simulink model "SdbTxSigProc"  (R2022b) (continued)
== system SdbTxSigProc == (continued)
  HvacAirTReAtFlrLeRaw_HvacAirTReAtFlrLeRaw/1 -> SdbTxSigProc_Runnable_sys/168(HvacAirTReAtFlrLeRaw_HvacAirTReAtFlrLeRaw_read)
  HvacAirTReAtVentLeRaw_HvacAirTReAtVentLeRaw/1 -> SdbTxSigProc_Runnable_sys/169(HvacAirTReAtVentLeRaw_HvacAirTReAtVentLeRaw_read)
  HvacEvaprTSpReNgcc_HvacEvaprTSpReNgcc/1 -> SdbTxSigProc_Runnable_sys/170(HvacEvaprTSpReNgcc_HvacEvaprTSpReNgcc_read)
  HvacFanLvlFbNgcc_HvacFanLvlFbNgcc/1 -> SdbTxSigProc_Runnable_sys/171(HvacFanLvlFbNgcc_HvacFanLvlFbNgcc_read)
  HvacFanLvlFbReNgcc_HvacFanLvlFbReNgcc/1 -> SdbTxSigProc_Runnable_sys/172(HvacFanLvlFbReNgcc_HvacFanLvlFbReNgcc_read)
  HvacHeatElmAirMFlowEstimdFirstLeNgcc_HvacHeatElmAirMFlowEstimdFirstLeNgcc/1 -> SdbTxSigProc_Runnable_sys/173(HvacHeatElmAirMFlowEstimdFirstLeNgcc_HvacHeatElmAirMFlowEstimdFirstLeNgcc_read)
  HvacHeatElmAirMFlowEstimdFirstRiNgcc_HvacHeatElmAirMFlowEstimdFirstRiNgcc/1 -> SdbTxSigProc_Runnable_sys/174(HvacHeatElmAirMFlowEstimdFirstRiNgcc_HvacHeatElmAirMFlowEstimdFirstRiNgcc_read)
  HvacHeatElmAirMFlowEstimdSecLeNgcc_HvacHeatElmAirMFlowEstimdSecLeNgcc/1 -> SdbTxSigProc_Runnable_sys/175(HvacHeatElmAirMFlowEstimdSecLeNgcc_HvacHeatElmAirMFlowEstimdSecLeNgcc_read)
  HvacHeatgReqReInt_HvacHeatgReqReInt/1 -> SdbTxSigProc_Runnable_sys/176(HvacHeatgReqReInt_HvacHeatgReqReInt_read)
  HvacHexAirTForRowFirstLeRaw_HvacHexAirTForRowFirstLeRaw/1 -> SdbTxSigProc_Runnable_sys/177(HvacHexAirTForRowFirstLeRaw_HvacHexAirTForRowFirstLeRaw_read)
  HvacHexAirTForRowFirstRiRaw_HvacHexAirTForRowFirstRiRaw/1 -> SdbTxSigProc_Runnable_sys/178(HvacHexAirTForRowFirstRiRaw_HvacHexAirTForRowFirstRiRaw_read)
  HvacHexAirTForRowSecLeRaw_HvacHexAirTForRowSecLeRaw/1 -> SdbTxSigProc_Runnable_sys/179(HvacHexAirTForRowSecLeRaw_HvacHexAirTForRowSecLeRaw_read)
  KeySynchrnztnLclNgcc_KeySynchrnztnLclNgcc/1 -> SdbTxSigProc_Runnable_sys/180(KeySynchrnztnLclNgcc_KeySynchrnztnLclNgcc_read)
  OutlSovActvStsNgcc_OutlSovActvStsNgcc/1 -> SdbTxSigProc_Runnable_sys/181(OutlSovActvStsNgcc_OutlSovActvStsNgcc_read)
  OxygenConcSnsrActnReqNgcc_OxygenConcSnsrActnReqNgcc/1 -> SdbTxSigProc_Runnable_sys/182(OxygenConcSnsrActnReqNgcc_OxygenConcSnsrActnReqNgcc_read)
  OxygenConcSnsrStsFrmClimaNgcc_OxygenConcSnsrStsFrmClimaNgcc/1 -> SdbTxSigProc_Runnable_sys/183(OxygenConcSnsrStsFrmClimaNgcc_OxygenConcSnsrStsFrmClimaNgcc_read)
  OxygenSplyAvlStsFrmClimaNgcc_OxygenSplyAvlStsFrmClimaNgcc/1 -> SdbTxSigProc_Runnable_sys/184(OxygenSplyAvlStsFrmClimaNgcc_OxygenSplyAvlStsFrmClimaNgcc_read)
  OxygenSplyErrFrmClimaNgcc_OxygenSplyErrFrmClimaNgcc/1 -> SdbTxSigProc_Runnable_sys/185(OxygenSplyErrFrmClimaNgcc_OxygenSplyErrFrmClimaNgcc_read)
  OxygenSplyPopUpReqNgcc_OxygenSplyPopUpReqNgcc/1 -> SdbTxSigProc_Runnable_sys/186(OxygenSplyPopUpReqNgcc_OxygenSplyPopUpReqNgcc_read)
  OxygenSplyPwrAllwdNgcc_OxygenSplyPwrAllwdNgcc/1 -> SdbTxSigProc_Runnable_sys/187(OxygenSplyPwrAllwdNgcc_OxygenSplyPwrAllwdNgcc_read)
  OxygenSplyReqFaildNgcc_OxygenSplyReqFaildNgcc/1 -> SdbTxSigProc_Runnable_sys/188(OxygenSplyReqFaildNgcc_OxygenSplyReqFaildNgcc_read)
  OxygenSplyReqNgcc_OxygenSplyReqNgcc/1 -> SdbTxSigProc_Runnable_sys/189(OxygenSplyReqNgcc_OxygenSplyReqNgcc_read)
  OxygenSplyVoiceFbNgcc_OxygenSplyVoiceFbNgcc/1 -> SdbTxSigProc_Runnable_sys/190(OxygenSplyVoiceFbNgcc_OxygenSplyVoiceFbNgcc_read)
  PrkgClimaEngStReqNgcc_PrkgClimaEngStReqNgcc/1 -> SdbTxSigProc_Runnable_sys/191(PrkgClimaEngStReqNgcc_PrkgClimaEngStReqNgcc_read)
  PwrCnsForHvacFanFrntNgcc_PwrCnsForHvacFanFrnt/1 -> SdbTxSigProc_Runnable_sys/192(PwrCnsForHvacFanFrntNgcc_PwrCnsForHvacFanFrnt_read)
  PwrCnsForHvacFanReNgcc_PwrCnsForHvacFanRe/1 -> SdbTxSigProc_Runnable_sys/193(PwrCnsForHvacFanReNgcc_PwrCnsForHvacFanRe_read)
  ReClimaManModCmftStsNgcc_ReClimaManModCmftStsNgcc/1 -> SdbTxSigProc_Runnable_sys/194(ReClimaManModCmftStsNgcc_ReClimaManModCmftStsNgcc_read)
  RemOxygenSplyActvFaildNgcc_RemOxygenSplyActvFaildNgcc/1 -> SdbTxSigProc_Runnable_sys/195(RemOxygenSplyActvFaildNgcc_RemOxygenSplyActvFaildNgcc_read)
  TCSCmprStrtFltNgcc_TCSCmprStrtFltNgcc/1 -> SdbTxSigProc_Runnable_sys/196(TCSCmprStrtFltNgcc_TCSCmprStrtFltNgcc_read)
  TCScoolgGrdReqNgcc_TCScoolgGrdReqNgcc/1 -> SdbTxSigProc_Runnable_sys/197(TCScoolgGrdReqNgcc_TCScoolgGrdReqNgcc_read)
  TCSCooltFlowReq1Ngcc_TCSCooltFlowReq1Ngcc/1 -> SdbTxSigProc_Runnable_sys/198(TCSCooltFlowReq1Ngcc_TCSCooltFlowReq1Ngcc_read)
  TCSCooltTReq1Ngcc_TCSCooltTReq1Ngcc/1 -> SdbTxSigProc_Runnable_sys/199(TCSCooltTReq1Ngcc_TCSCooltTReq1Ngcc_read)
  TCSDoorStsNgcc_TCSDoorStsNgcc/1 -> SdbTxSigProc_Runnable_sys/200(TCSDoorStsNgcc_TCSDoorStsNgcc_read)
  TCSDoorTopSts1Ngcc_TCSDoorTopSts1Ngcc/1 -> SdbTxSigProc_Runnable_sys/201(TCSDoorTopSts1Ngcc_TCSDoorTopSts1Ngcc_read)
  TcsEcoModSts1Ngcc_TcsEcoModSts1Ngcc/1 -> SdbTxSigProc_Runnable_sys/202(TcsEcoModSts1Ngcc_TcsEcoModSts1Ngcc_read)
  TcsFltNewSts1Ngcc_TcsFltNewSts1Ngcc/1 -> SdbTxSigProc_Runnable_sys/203(TcsFltNewSts1Ngcc_TcsFltNewSts1Ngcc_read)
  TCSFltStsNgcc_TCSFltStsNgcc/1 -> SdbTxSigProc_Runnable_sys/204(TCSFltStsNgcc_TCSFltStsNgcc_read)
  TCSModSwtStsNgcc_TCSModSwtStsNgcc/1 -> SdbTxSigProc_Runnable_sys/205(TCSModSwtStsNgcc_TCSModSwtStsNgcc_read)
  TCSnsrTStsNgcc_TCSnsrTStsNgcc/1 -> SdbTxSigProc_Runnable_sys/206(TCSnsrTStsNgcc_TCSnsrTStsNgcc_read)
  TCSRadrTreqNgcc_TCSRadrTreqNgcc/1 -> SdbTxSigProc_Runnable_sys/207(TCSRadrTreqNgcc_TCSRadrTreqNgcc_read)
  TCSRadrTStsNgcc_TCSRadrTStsNgcc/1 -> SdbTxSigProc_Runnable_sys/208(TCSRadrTStsNgcc_TCSRadrTStsNgcc_read)
  TCSTmrOverStsNgcc_TCSTmrOverStsNgcc/1 -> SdbTxSigProc_Runnable_sys/209(TCSTmrOverStsNgcc_TCSTmrOverStsNgcc_read)
  TCSTmrStrtStsNgcc_TCSTmrStrtStsNgcc/1 -> SdbTxSigProc_Runnable_sys/210(TCSTmrStrtStsNgcc_TCSTmrStrtStsNgcc_read)
  TCSTNowStsNgcc_TCSTNowStsNgcc/1 -> SdbTxSigProc_Runnable_sys/211(TCSTNowStsNgcc_TCSTNowStsNgcc_read)
  TgtHvacAirTForMaxTZoneNgcc_TgtHvacAirTForMaxTZoneNgcc/1 -> SdbTxSigProc_Runnable_sys/212(TgtHvacAirTForMaxTZoneNgcc_TgtHvacAirTForMaxTZoneNgcc_read)
  TgtHvacAirTForRowFirstLeNgcc_TgtHvacAirTForRowFirstLeNgcc/1 -> SdbTxSigProc_Runnable_sys/213(TgtHvacAirTForRowFirstLeNgcc_TgtHvacAirTForRowFirstLeNgcc_read)
  TgtHvacAirTForRowFirstRiNgcc_TgtHvacAirTForRowFirstRiNgcc/1 -> SdbTxSigProc_Runnable_sys/214(TgtHvacAirTForRowFirstRiNgcc_TgtHvacAirTForRowFirstRiNgcc_read)
  TgtHvacAirTForRowSecLeNgcc_TgtHvacAirTForRowSecLeNgcc/1 -> SdbTxSigProc_Runnable_sys/215(TgtHvacAirTForRowSecLeNgcc_TgtHvacAirTForRowSecLeNgcc_read)
  TgtHvacAirTForRowSecRiNgcc_TgtHvacAirTForRowSecRiNgcc/1 -> SdbTxSigProc_Runnable_sys/216(TgtHvacAirTForRowSecRiNgcc_TgtHvacAirTForRowSecRiNgcc_read)
  ThermoTSteDeoSts1Ngcc_ThermoTSteDeoSts1Ngcc/1 -> SdbTxSigProc_Runnable_sys/217(ThermoTSteDeoSts1Ngcc_ThermoTSteDeoSts1Ngcc_read)
  ThrdRowLeSeatHeatgPwrAllwdNgcc_ThrdRowLeSeatHeatgPwrAllwdNgcc/1 -> SdbTxSigProc_Runnable_sys/218(ThrdRowLeSeatHeatgPwrAllwdNgcc_ThrdRowLeSeatHeatgPwrAllwdNgcc_read)
  ThrdRowLeSeatVentnPwrAllwdNgcc_ThrdRowLeSeatVentnPwrAllwdNgcc/1 -> SdbTxSigProc_Runnable_sys/219(ThrdRowLeSeatVentnPwrAllwdNgcc_ThrdRowLeSeatVentnPwrAllwdNgcc_read)
  ThrdRowRiSeatHeatgPwrAllwdNgcc_ThrdRowRiSeatHeatgPwrAllwdNgcc/1 -> SdbTxSigProc_Runnable_sys/220(ThrdRowRiSeatHeatgPwrAllwdNgcc_ThrdRowRiSeatHeatgPwrAllwdNgcc_read)
  ThrdRowRiSeatVentnPwrAllwdNgcc_ThrdRowRiSeatVentnPwrAllwdNgcc/1 -> SdbTxSigProc_Runnable_sys/221(ThrdRowRiSeatVentnPwrAllwdNgcc_ThrdRowRiSeatVentnPwrAllwdNgcc_read)
  TiOfDeactvnForClimaCtrlNgcc_TiOfDeactvnForClimaCtrl/1 -> SdbTxSigProc_Runnable_sys/222(TiOfDeactvnForClimaCtrlNgcc_TiOfDeactvnForClimaCtrl_read)
  VMMChngNdMtnNgcc_VMMChngNdMtnNgcc/1 -> SdbTxSigProc_Runnable_sys/223(VMMChngNdMtnNgcc_VMMChngNdMtnNgcc_read)
  SdbTxSigProc_Runnable_sys/1(AACAirClngActn_AACAirClngActn_write) -> AACAirClngActn_AACAirClngActn/1
  SdbTxSigProc_Runnable_sys/2(AirSrcReq_AirSrcReq_write) -> AirSrcReq_AirSrcReq/1
  SdbTxSigProc_Runnable_sys/3(AmbTFildForClima_AmbTFildForClima_write) -> AmbTFildForClima_AmbTFildForClima/1
  SdbTxSigProc_Runnable_sys/4(AutOxygenSplyStsFrmClima_AutOxygenSplyStsFrmClima_write) -> AutOxygenSplyStsFrmClima_AutOxygenSplyStsFrmClima/1
  SdbTxSigProc_Runnable_sys/5(CcmAACAirClngSts_CcmAACAirClngSts_write) -> CcmAACAirClngSts_CcmAACAirClngSts/1
  SdbTxSigProc_Runnable_sys/6(CCMDataTraceZCUP1_CCMDataTraceZCUP1_write) -> CCMDataTraceZCUP1_CCMDataTraceZCUP1/1
  SdbTxSigProc_Runnable_sys/7(CCMDataTraceZCUP2_CCMDataTraceZCUP2_write) -> CCMDataTraceZCUP2_CCMDataTraceZCUP2/1
  SdbTxSigProc_Runnable_sys/8(CCMDataTraceZCUP3_CCMDataTraceZCUP3_write) -> CCMDataTraceZCUP3_CCMDataTraceZCUP3/1
  SdbTxSigProc_Runnable_sys/9(CCMDataTraceZCUP4_CCMDataTraceZCUP4_write) -> CCMDataTraceZCUP4_CCMDataTraceZCUP4/1
  SdbTxSigProc_Runnable_sys/10(CCMDataTraceZCUP5_CCMDataTraceZCUP5_write) -> CCMDataTraceZCUP5_CCMDataTraceZCUP5/1
  SdbTxSigProc_Runnable_sys/11(CCMDataTraceZCUP6_CCMDataTraceZCUP6_write) -> CCMDataTraceZCUP6_CCMDataTraceZCUP6/1
  SdbTxSigProc_Runnable_sys/12(CCMDataTraceZCUP7_CCMDataTraceZCUP7_write) -> CCMDataTraceZCUP7_CCMDataTraceZCUP7/1
  SdbTxSigProc_Runnable_sys/13(CcmElecAirDirCrtlReqSecCnsLePosX_CcmElecAirDirCrtlReqSecCnsLePosX_write) -> CcmElecAirDirCrtlReqSecCnsLePosX_CcmElecAirDirCrtlReqSecCnsLePosX/1
  SdbTxSigProc_Runnable_sys/14(CcmElecAirDirCrtlReqSecCnsLePosY_CcmElecAirDirCrtlReqSecCnsLePosY_write) -> CcmElecAirDirCrtlReqSecCnsLePosY_CcmElecAirDirCrtlReqSecCnsLePosY/1
  SdbTxSigProc_Runnable_sys/15(CcmElecAirDirCrtlReqSecCnsRiPosX_CcmElecAirDirCrtlReqSecCnsRiPosX_write) -> CcmElecAirDirCrtlReqSecCnsRiPosX_CcmElecAirDirCrtlReqSecCnsRiPosX/1
  SdbTxSigProc_Runnable_sys/16(CcmElecAirDirCrtlReqSecCnsRiPosY_CcmElecAirDirCrtlReqSecCnsRiPosY_write) -> CcmElecAirDirCrtlReqSecCnsRiPosY_CcmElecAirDirCrtlReqSecCnsRiPosY/1
  SdbTxSigProc_Runnable_sys/17(CCMFragCh1OvrdDy_CCMFragCh1OvrdDy_write) -> CCMFragCh1OvrdDy_CCMFragCh1OvrdDy/1
  SdbTxSigProc_Runnable_sys/18(CCMFragCh2OvrdDy_CCMFragCh2OvrdDy_write) -> CCMFragCh2OvrdDy_CCMFragCh2OvrdDy/1
  SdbTxSigProc_Runnable_sys/19(CCMFragCh3OvrdDy_CCMFragCh3OvrdDy_write) -> CCMFragCh3OvrdDy_CCMFragCh3OvrdDy/1
  SdbTxSigProc_Runnable_sys/20(CCMFragCh4OvrdDy_CCMFragCh4OvrdDy_write) -> CCMFragCh4OvrdDy_CCMFragCh4OvrdDy/1
  SdbTxSigProc_Runnable_sys/21(CCMFragCh5OvrdDy_CCMFragCh5OvrdDy_write) -> CCMFragCh5OvrdDy_CCMFragCh5OvrdDy/1
  SdbTxSigProc_Runnable_sys/22(CCMFragraCh1AvlTiPrec_CCMFragraCh1AvlTiPrec_write) -> CCMFragraCh1AvlTiPrec_CCMFragraCh1AvlTiPrec/1
  SdbTxSigProc_Runnable_sys/23(CCMFragraCh2AvlTiPrec_CCMFragraCh2AvlTiPrec_write) -> CCMFragraCh2AvlTiPrec_CCMFragraCh2AvlTiPrec/1
  SdbTxSigProc_Runnable_sys/24(CCMFragraCh3AvlTiPrec_CCMFragraCh3AvlTiPrec_write) -> CCMFragraCh3AvlTiPrec_CCMFragraCh3AvlTiPrec/1
  SdbTxSigProc_Runnable_sys/25(CCMFragraCh4AvlTiPrec_CCMFragraCh4AvlTiPrec_write) -> CCMFragraCh4AvlTiPrec_CCMFragraCh4AvlTiPrec/1
  SdbTxSigProc_Runnable_sys/26(CCMFragraCh5AvlTiPrec_CCMFragraCh5AvlTiPrec_write) -> CCMFragraCh5AvlTiPrec_CCMFragraCh5AvlTiPrec/1
  SdbTxSigProc_Runnable_sys/27(CcmWbmMasterReqLeLin1_CcmWbmMasterReqLeLin1_write) -> CcmWbmMasterReqLeLin1_CcmWbmMasterReqLeLin1/1
  SdbTxSigProc_Runnable_sys/28(CcmWbmMasterReqRiLin1_CcmWbmMasterReqRiLin1_write) -> CcmWbmMasterReqRiLin1_CcmWbmMasterReqRiLin1/1
  SdbTxSigProc_Runnable_sys/29(ChgFilElmRst_ChgFilElmRst_write) -> ChgFilElmRst_ChgFilElmRst/1
  SdbTxSigProc_Runnable_sys/30(ClimaAtRowSecActv_ClimaAtRowSecActv_write) -> ClimaAtRowSecActv_ClimaAtRowSecActv/1
  SdbTxSigProc_Runnable_sys/31(ClimaCtrlActv_ClimaCtrlActv_write) -> ClimaCtrlActv_ClimaCtrlActv/1
  SdbTxSigProc_Runnable_sys/32(ClimaCtrlTiOfDeactvn_ClimaCtrlTiOfDeactvn_write) -> ClimaCtrlTiOfDeactvn_ClimaCtrlTiOfDeactvn/1
  SdbTxSigProc_Runnable_sys/33(ClimaEstimdTForHeat_ClimaEstimdTForHeat_write) -> ClimaEstimdTForHeat_ClimaEstimdTForHeat/1
  SdbTxSigProc_Runnable_sys/34(ClimaEstimdTForReHeat_ClimaEstimdTForReHeat_write) -> ClimaEstimdTForReHeat_ClimaEstimdTForReHeat/1
  SdbTxSigProc_Runnable_sys/35(ClimaManModCmftPopUpReq_ClimaManModCmftPopUpReq_write) -> ClimaManModCmftPopUpReq_ClimaManModCmftPopUpReq/1
  SdbTxSigProc_Runnable_sys/36(CmptmtAirDistbnRsp_CmptmtAirDistbnRsp_write) -> CmptmtAirDistbnRsp_CmptmtAirDistbnRsp/1
  SdbTxSigProc_Runnable_sys/37(CmptmtOxygenConcLvlFrmClima_CmptmtOxygenConcLvlFrmClima_write) -> CmptmtOxygenConcLvlFrmClima_CmptmtOxygenConcLvlFrmClima/1
  SdbTxSigProc_Runnable_sys/38(CmptmtOxygenConcVluFrmClima_CmptmtOxygenConcVluFrmClima_write) -> CmptmtOxygenConcVluFrmClima_CmptmtOxygenConcVluFrmClima/1
  SdbTxSigProc_Runnable_sys/39(CoupldCtrlErrAtRowFirstLe_CoupldCtrlErrAtRowFirstLe_write) -> CoupldCtrlErrAtRowFirstLe_CoupldCtrlErrAtRowFirstLe/1
  SdbTxSigProc_Runnable_sys/40(CoupldCtrlErrAtRowFirstRi_CoupldCtrlErrAtRowFirstRi_write) -> CoupldCtrlErrAtRowFirstRi_CoupldCtrlErrAtRowFirstRi/1
  SdbTxSigProc_Runnable_sys/41(CoupldCtrlErrAtRowSecLe_CoupldCtrlErrAtRowSecLe_write) -> CoupldCtrlErrAtRowSecLe_CoupldCtrlErrAtRowSecLe/1
  SdbTxSigProc_Runnable_sys/42(CoupldCtrlErrAtRowSecRi_CoupldCtrlErrAtRowSecRi_write) -> CoupldCtrlErrAtRowSecRi_CoupldCtrlErrAtRowSecRi/1
  SdbTxSigProc_Runnable_sys/43(FrntBlwSpdReq_FrntBlwSpdReq_write) -> FrntBlwSpdReq_FrntBlwSpdReq/1
  SdbTxSigProc_Runnable_sys/44(FrntClimaManModCmftSts_FrntClimaManModCmftSts_write) -> FrntClimaManModCmftSts_FrntClimaManModCmftSts/1
  SdbTxSigProc_Runnable_sys/45(HeatrAirTReqFirstLe_HeatrAirTReqFirstLe_write) -> HeatrAirTReqFirstLe_HeatrAirTReqFirstLe/1
  SdbTxSigProc_Runnable_sys/46(HeatrAirTReqFirstRi_HeatrAirTReqFirstRi_write) -> HeatrAirTReqFirstRi_HeatrAirTReqFirstRi/1
  SdbTxSigProc_Runnable_sys/47(HeatrAirTReqSecLe_HeatrAirTReqSecLe_write) -> HeatrAirTReqSecLe_HeatrAirTReqSecLe/1
  SdbTxSigProc_Runnable_sys/48(HighTempCleanRunningSts_HighTempCleanRunningSts_write) -> HighTempCleanRunningSts_HighTempCleanRunningSts/1
  SdbTxSigProc_Runnable_sys/49(HighTempCleanSettingSts_HighTempCleanSettingSts_write) -> HighTempCleanSettingSts_HighTempCleanSettingSts/1
  SdbTxSigProc_Runnable_sys/50(HvacAirTForRowThrdAtFlrRi_HvacAirTForRowThrdAtFlrRi_write) -> HvacAirTForRowThrdAtFlrRi_HvacAirTForRowThrdAtFlrRi/1
  SdbTxSigProc_Runnable_sys/51(HvacAirTForRowThrdAtVentRi_HvacAirTForRowThrdAtVentRi_write) -> HvacAirTForRowThrdAtVentRi_HvacAirTForRowThrdAtVentRi/1
  SdbTxSigProc_Runnable_sys/52(HvacEvaprTSpRe_HvacEvaprTSpRe_write) -> HvacEvaprTSpRe_HvacEvaprTSpRe/1
  SdbTxSigProc_Runnable_sys/53(HvacFanLvlFbFrnt_HvacFanLvlFbFrnt_write) -> HvacFanLvlFbFrnt_HvacFanLvlFbFrnt/1
  SdbTxSigProc_Runnable_sys/54(HvacFanLvlFbRe_HvacFanLvlFbRe_write) -> HvacFanLvlFbRe_HvacFanLvlFbRe/1
  SdbTxSigProc_Runnable_sys/55(HvacHeatElmAirMFlowEstimdFirstLe_HvacHeatElmAirMFlowEstimdFirstLe_write) -> HvacHeatElmAirMFlowEstimdFirstLe_HvacHeatElmAirMFlowEstimdFirstLe/1
  SdbTxSigProc_Runnable_sys/56(HvacHeatElmAirMFlowEstimdFirstRi_HvacHeatElmAirMFlowEstimdFirstRi_write) -> HvacHeatElmAirMFlowEstimdFirstRi_HvacHeatElmAirMFlowEstimdFirstRi/1
  SdbTxSigProc_Runnable_sys/57(HvacHeatElmAirMFlowEstimdSecLe_HvacHeatElmAirMFlowEstimdSecLe_write) -> HvacHeatElmAirMFlowEstimdSecLe_HvacHeatElmAirMFlowEstimdSecLe/1
  SdbTxSigProc_Runnable_sys/58(HvacHexAirTFirstLe_HvacHexAirTFirstLe_write) -> HvacHexAirTFirstLe_HvacHexAirTFirstLe/1
  SdbTxSigProc_Runnable_sys/59(HvacHexAirTFirstRi_HvacHexAirTFirstRi_write) -> HvacHexAirTFirstRi_HvacHexAirTFirstRi/1
  SdbTxSigProc_Runnable_sys/60(HvacHexAirTSecLe_HvacHexAirTSecLe_write) -> HvacHexAirTSecLe_HvacHexAirTSecLe/1
  SdbTxSigProc_Runnable_sys/61(HvahAirTsp_HvahAirTsp_write) -> HvahAirTsp_HvahAirTsp/1
  SdbTxSigProc_Runnable_sys/62(HvahHeatgReq_HvahHeatgReq_write) -> HvahHeatgReq_HvahHeatgReq/1
  SdbTxSigProc_Runnable_sys/63(HwFrntBlwPwmReq_HwFrntBlwPwmReq_write) -> HwFrntBlwPwmReq_HwFrntBlwPwmReq/1
  SdbTxSigProc_Runnable_sys/64(KeySynchrnztnLcl_KeySynchrnztnLcl_write) -> KeySynchrnztnLcl_KeySynchrnztnLcl/1
  SdbTxSigProc_Runnable_sys/65(MistRiskLvl_MistRiskLvl_write) -> MistRiskLvl_MistRiskLvl/1
  SdbTxSigProc_Runnable_sys/66(OutlSovActvSts_OutlSovActvSts_write) -> OutlSovActvSts_OutlSovActvSts/1
  SdbTxSigProc_Runnable_sys/67(OxygenConcSnsrActnReq_OxygenConcSnsrActnReq_write) -> OxygenConcSnsrActnReq_OxygenConcSnsrActnReq/1
  SdbTxSigProc_Runnable_sys/68(OxygenConcSnsrStsFrmClima_OxygenConcSnsrStsFrmClima_write) -> OxygenConcSnsrStsFrmClima_OxygenConcSnsrStsFrmClima/1
  SdbTxSigProc_Runnable_sys/69(OxygenSplyAvlStsFrmClima_OxygenSplyAvlStsFrmClima_write) -> OxygenSplyAvlStsFrmClima_OxygenSplyAvlStsFrmClima/1
  SdbTxSigProc_Runnable_sys/70(OxygenSplyErrFrmClima_OxygenSplyErrFrmClima_write) -> OxygenSplyErrFrmClima_OxygenSplyErrFrmClima/1
  SdbTxSigProc_Runnable_sys/71(OxygenSplyPopUpReq_OxygenSplyPopUpReq_write) -> OxygenSplyPopUpReq_OxygenSplyPopUpReq/1
  SdbTxSigProc_Runnable_sys/72(OxygenSplyPwrAllwd_OxygenSplyPwrAllwd_write) -> OxygenSplyPwrAllwd_OxygenSplyPwrAllwd/1
  SdbTxSigProc_Runnable_sys/73(OxygenSplyReq_OxygenSplyReq_write) -> OxygenSplyReq_OxygenSplyReq/1
  SdbTxSigProc_Runnable_sys/74(OxygenSplyReqFaild_OxygenSplyReqFaild_write) -> OxygenSplyReqFaild_OxygenSplyReqFaild/1
  SdbTxSigProc_Runnable_sys/75(OxygenSplyVoiceFb_OxygenSplyVoiceFb_write) -> OxygenSplyVoiceFb_OxygenSplyVoiceFb/1
  SdbTxSigProc_Runnable_sys/76(PrkgClimaEngStReq_PrkgClimaEngStReq_write) -> PrkgClimaEngStReq_PrkgClimaEngStReq/1
  SdbTxSigProc_Runnable_sys/77(PwrCnsForHvacFanFrnt_PwrCnsForHvacFanFrnt_write) -> PwrCnsForHvacFanFrnt_PwrCnsForHvacFanFrnt/1
  SdbTxSigProc_Runnable_sys/78(PwrCnsForHvacFanRe_PwrCnsForHvacFanRe_write) -> PwrCnsForHvacFanRe_PwrCnsForHvacFanRe/1
  SdbTxSigProc_Runnable_sys/79(ReClimaManModCmftSts_ReClimaManModCmftSts_write) -> ReClimaManModCmftSts_ReClimaManModCmftSts/1
  SdbTxSigProc_Runnable_sys/80(RemClimaElecDefrstReq_RemClimaElecDefrstReq_write) -> RemClimaElecDefrstReq_RemClimaElecDefrstReq/1
  SdbTxSigProc_Runnable_sys/81(RemOxygenSplyActvFaild_RemOxygenSplyActvFaild_write) -> RemOxygenSplyActvFaild_RemOxygenSplyActvFaild/1
  SdbTxSigProc_Runnable_sys/82(TCSCmprStrtFlt_TCSCmprStrtFlt_write) -> TCSCmprStrtFlt_TCSCmprStrtFlt/1
  SdbTxSigProc_Runnable_sys/83(TCSCmprStrtFlt1_TCSCmprStrtFlt1_write) -> TCSCmprStrtFlt1_TCSCmprStrtFlt1/1
  SdbTxSigProc_Runnable_sys/84(TCScoolgGrdReq_TCScoolgGrdReq_write) -> TCScoolgGrdReq_TCScoolgGrdReq/1
  SdbTxSigProc_Runnable_sys/85(TCScoolgGrdReq1_TCScoolgGrdReq1_write) -> TCScoolgGrdReq1_TCScoolgGrdReq1/1
  SdbTxSigProc_Runnable_sys/86(TCSCooltFlowReq1_TCSCooltFlowReq1_write) -> TCSCooltFlowReq1_TCSCooltFlowReq1/1
  SdbTxSigProc_Runnable_sys/87(TCSCooltTReq1_TCSCooltTReq1_write) -> TCSCooltTReq1_TCSCooltTReq1/1
  SdbTxSigProc_Runnable_sys/88(TCSDoorSts_TCSDoorSts_write) -> TCSDoorSts_TCSDoorSts/1
  SdbTxSigProc_Runnable_sys/89(TCSDoorSts1_TCSDoorSts1_write) -> TCSDoorSts1_TCSDoorSts1/1
  SdbTxSigProc_Runnable_sys/90(TCSDoorTopSts1_TCSDoorTopSts1_write) -> TCSDoorTopSts1_TCSDoorTopSts1/1
  SdbTxSigProc_Runnable_sys/91(TcsEcoModSts1_Element_write) -> TcsEcoModSts1_Element/1
  SdbTxSigProc_Runnable_sys/92(TcsFltNewSts1_TcsFltNewSts1_write) -> TcsFltNewSts1_TcsFltNewSts1/1
  SdbTxSigProc_Runnable_sys/93(TCSFltSts_TCSFltSts_write) -> TCSFltSts_TCSFltSts/1
  SdbTxSigProc_Runnable_sys/94(TCSFltSts1_TCSFltSts1_write) -> TCSFltSts1_TCSFltSts1/1
  SdbTxSigProc_Runnable_sys/95(TCSModSwtSts_TCSModSwtSts_write) -> TCSModSwtSts_TCSModSwtSts/1
  SdbTxSigProc_Runnable_sys/96(TCSModSwtSts1_TCSModSwtSts1_write) -> TCSModSwtSts1_TCSModSwtSts1/1
  SdbTxSigProc_Runnable_sys/97(TCSnsrTSts_TCSnsrTSts_write) -> TCSnsrTSts_TCSnsrTSts/1
  SdbTxSigProc_Runnable_sys/98(TCSnsrTSts1_TCSnsrTSts1_write) -> TCSnsrTSts1_TCSnsrTSts1/1
  SdbTxSigProc_Runnable_sys/99(TCSRadrTreq_TCSRadrTreq_write) -> TCSRadrTreq_TCSRadrTreq/1
  SdbTxSigProc_Runnable_sys/100(TCSRadrTreq1_TCSRadrTreq1_write) -> TCSRadrTreq1_TCSRadrTreq1/1
  SdbTxSigProc_Runnable_sys/101(TCSRadrTSts_TCSRadrTSts_write) -> TCSRadrTSts_TCSRadrTSts/1
  SdbTxSigProc_Runnable_sys/102(TCSRadrTSts1_TCSRadrTSts1_write) -> TCSRadrTSts1_TCSRadrTSts1/1
  SdbTxSigProc_Runnable_sys/103(TCSTmrOverSts_TCSTmrOverSts_write) -> TCSTmrOverSts_TCSTmrOverSts/1
  SdbTxSigProc_Runnable_sys/104(TCSTmrOverSts1_TCSTmrOverSts1_write) -> TCSTmrOverSts1_TCSTmrOverSts1/1
  SdbTxSigProc_Runnable_sys/105(TCSTmrStrtSts_TCSTmrStrtSts_write) -> TCSTmrStrtSts_TCSTmrStrtSts/1
  SdbTxSigProc_Runnable_sys/106(TCSTmrStrtSts1_TCSTmrStrtSts1_write) -> TCSTmrStrtSts1_TCSTmrStrtSts1/1
  SdbTxSigProc_Runnable_sys/107(TCSTNowSts_TCSTNowSts_write) -> TCSTNowSts_TCSTNowSts/1
  SdbTxSigProc_Runnable_sys/108(TCSTNowSts1_TCSTNowSts1_write) -> TCSTNowSts1_TCSTNowSts1/1
  SdbTxSigProc_Runnable_sys/109(TgtHvacAirTForMaxTZone_TgtHvacAirTForMaxTZone_write) -> TgtHvacAirTForMaxTZone_TgtHvacAirTForMaxTZone/1
  SdbTxSigProc_Runnable_sys/110(ThermoTSteDeoSts1_ThermoTSteDeoSts1_write) -> ThermoTSteDeoSts1_ThermoTSteDeoSts1/1
  SdbTxSigProc_Runnable_sys/111(ThrdRowLeSeatHeatgPwrAllwd_ThrdRowLeSeatHeatgPwrAllwd_write) -> ThrdRowLeSeatHeatgPwrAllwd_ThrdRowLeSeatHeatgPwrAllwd/1
  SdbTxSigProc_Runnable_sys/112(ThrdRowLeSeatVentnPwrAllwd_ThrdRowLeSeatVentnPwrAllwd_write) -> ThrdRowLeSeatVentnPwrAllwd_ThrdRowLeSeatVentnPwrAllwd/1
  SdbTxSigProc_Runnable_sys/113(ThrdRowRiSeatHeatgPwrAllwd_ThrdRowRiSeatHeatgPwrAllwd_write) -> ThrdRowRiSeatHeatgPwrAllwd_ThrdRowRiSeatHeatgPwrAllwd/1
  SdbTxSigProc_Runnable_sys/114(ThrdRowRiSeatVentnPwrAllwd_ThrdRowRiSeatVentnPwrAllwd_write) -> ThrdRowRiSeatVentnPwrAllwd_ThrdRowRiSeatVentnPwrAllwd/1
  SdbTxSigProc_Runnable_sys/115(TiOfDeactvnForClimaCtrl_TiOfDeactvnForClimaCtrl_write) -> TiOfDeactvnForClimaCtrl_TiOfDeactvnForClimaCtrl/1
  SdbTxSigProc_Runnable_sys/116(VMMChngNdMtn_VMMChngNdMtn_write) -> VMMChngNdMtn_VMMChngNdMtn/1
  SdbTxSigProc_Runnable_sys/117(AirTFbFromClima_AirTFbFromClima_write) -> AirTFbFromClima_AirTFbFromClima/1
  AmbTEstimd_AmbTEstimd_merge/1 -> AmbTEstimd_AmbTEstimd/1
  SdbTxSigProc_Runnable_sys/119(CarTiIntForClima_CarTiIntForClima_write) -> CarTiIntForClima_CarTiIntForClima/1
  CcmCaliSig1_CcmCaliSig1_merge/1 -> CcmCaliSig1_CcmCaliSig1/1
  CcmCaliSig10_CcmCaliSig10_merge/1 -> CcmCaliSig10_CcmCaliSig10/1
  CcmCaliSig11_CcmCaliSig11_merge/1 -> CcmCaliSig11_CcmCaliSig11/1
  CcmCaliSig12_CcmCaliSig12_merge/1 -> CcmCaliSig12_CcmCaliSig12/1
  CcmCaliSig2_CcmCaliSig2_merge/1 -> CcmCaliSig2_CcmCaliSig2/1
  CcmCaliSig3_CcmCaliSig3_merge/1 -> CcmCaliSig3_CcmCaliSig3/1
  CcmCaliSig4_CcmCaliSig4_merge/1 -> CcmCaliSig4_CcmCaliSig4/1
  CcmCaliSig5_CcmCaliSig5_merge/1 -> CcmCaliSig5_CcmCaliSig5/1
  CcmCaliSig6_CcmCaliSig6_merge/1 -> CcmCaliSig6_CcmCaliSig6/1
  CcmCaliSig7_CcmCaliSig7_merge/1 -> CcmCaliSig7_CcmCaliSig7/1
  CcmCaliSig8_CcmCaliSig8_merge/1 -> CcmCaliSig8_CcmCaliSig8/1
  CcmCaliSig9_CcmCaliSig9_merge/1 -> CcmCaliSig9_CcmCaliSig9/1
  SdbTxSigProc_Runnable_sys/132(ClimaActv_ClimaActv_write) -> ClimaActv_ClimaActv/1
  SdbTxSigProc_Runnable_sys/133(ClimaActvAlrmSts_ClimaActvAlrmSts_write) -> ClimaActvAlrmSts_ClimaActvAlrmSts/1
  SdbTxSigProc_Runnable_sys/134(ClimaCmptSts_ClimaCmptSts_write) -> ClimaCmptSts_ClimaCmptSts/1
  SdbTxSigProc_Runnable_sys/135(ClimaDefrstSts_ClimaDefrstSts_write) -> ClimaDefrstSts_ClimaDefrstSts/1
  SdbTxSigProc_Runnable_sys/136(ClimaOffReq_ClimaOffReq_write) -> ClimaOffReq_ClimaOffReq/1
  SdbTxSigProc_Runnable_sys/137(ClimaPwrCns_ClimaPwrCns_write) -> ClimaPwrCns_ClimaPwrCns/1
  SdbTxSigProc_Runnable_sys/138(ClimaPwrCritDes_ClimaPwrCritDes_write) -> ClimaPwrCritDes_ClimaPwrCritDes/1
  SdbTxSigProc_Runnable_sys/139(ClimaPwrNormDes_ClimaPwrNormDes_write) -> ClimaPwrNormDes_ClimaPwrNormDes/1
  SdbTxSigProc_Runnable_sys/140(ClimaTiOfEnd_ClimaTiOfEnd_write) -> ClimaTiOfEnd_ClimaTiOfEnd/1
  SdbTxSigProc_Runnable_sys/141(CmptModForHp_CmptModForHp_write) -> CmptModForHp_CmptModForHp/1
  CmptmtAirTEstimdAtRowFirstLoResl_CmptmtAirTEstimdAtRowFirstLoResl_merge/1 -> CmptmtAirTEstimdAtRowFirstLoResl_CmptmtAirTEstimdAtRowFirstLoResl/1
  CmptmtAirTEstimdExtd_CmptmtAirTEstimdExtd_merge/1 -> CmptmtAirTEstimdExtd_CmptmtAirTEstimdExtd/1
  SdbTxSigProc_Runnable_sys/144(CupHoldrActvAllwd_CupHoldrActvAllwd_write) -> CupHoldrActvAllwd_CupHoldrActvAllwd/1
  SdbTxSigProc_Runnable_sys/145(DefrstMaxSts_DefrstMaxSts_write) -> DefrstMaxSts_DefrstMaxSts/1
  SdbTxSigProc_Runnable_sys/146(DrvrSeatHeatgPwrAllwd_DrvrSeatHeatgPwrAllwd_write) -> DrvrSeatHeatgPwrAllwd_DrvrSeatHeatgPwrAllwd/1
  SdbTxSigProc_Runnable_sys/147(DrvrSeatVentnPwrAllwd_DrvrSeatVentnPwrAllwd_write) -> DrvrSeatVentnPwrAllwd_DrvrSeatVentnPwrAllwd/1
  SdbTxSigProc_Runnable_sys/148(EcoClimaSts_EcoClimaSts_write) -> EcoClimaSts_EcoClimaSts/1
  SdbTxSigProc_Runnable_sys/149(EgyDesForClima_EgyDesForClima_write) -> EgyDesForClima_EgyDesForClima/1
  SdbTxSigProc_Runnable_sys/150(ElecDefrstReq_ElecDefrstReq_write) -> ElecDefrstReq_ElecDefrstReq/1
  SdbTxSigProc_Runnable_sys/151(EngRunngReqByClima_EngRunngReqByClima_write) -> EngRunngReqByClima_EngRunngReqByClima/1
  SdbTxSigProc_Runnable_sys/152(EngSpdIncIdleForClima_EngSpdIncIdleForClima_write) -> EngSpdIncIdleForClima_EngSpdIncIdleForClima/1
  SdbTxSigProc_Runnable_sys/153(EvaprTFrnt_EvaprTFrnt_write) -> EvaprTFrnt_EvaprTFrnt/1
  SdbTxSigProc_Runnable_sys/154(ExtVentToastFrmClima_ExtVentToastFrmClima_write) -> ExtVentToastFrmClima_ExtVentToastFrmClima/1
  HeatrAirTReq_HeatrAirTReq_merge/1 -> HeatrAirTReq_HeatrAirTReq/1
  SdbTxSigProc_Runnable_sys/156(HvacAirMFlowEstimd_HvacAirMFlowEstimd_write) -> HvacAirMFlowEstimd_HvacAirMFlowEstimd/1
  SdbTxSigProc_Runnable_sys/157(HvacAirMFlowReEstimd_HvacAirMFlowReEstimd_write) -> HvacAirMFlowReEstimd_HvacAirMFlowReEstimd/1
  SdbTxSigProc_Runnable_sys/158(HvacAirMFlowVent_HvacAirMFlowVent_write) -> HvacAirMFlowVent_HvacAirMFlowVent/1
  SdbTxSigProc_Runnable_sys/159(HvacAirTForRowFirstAtFlrLe_HvacAirTForRowFirstAtFlrLe_write) -> HvacAirTForRowFirstAtFlrLe_HvacAirTForRowFirstAtFlrLe/1
  SdbTxSigProc_Runnable_sys/160(HvacAirTForRowFirstAtFlrRi_HvacAirTForRowFirstAtFlrRi_write) -> HvacAirTForRowFirstAtFlrRi_HvacAirTForRowFirstAtFlrRi/1
  SdbTxSigProc_Runnable_sys/161(HvacAirTForRowFirstAtVentLe_HvacAirTForRowFirstAtVentLe_write) -> HvacAirTForRowFirstAtVentLe_HvacAirTForRowFirstAtVentLe/1
  SdbTxSigProc_Runnable_sys/162(HvacAirTForRowFirstAtVentRi_HvacAirTForRowFirstAtVentRi_write) -> HvacAirTForRowFirstAtVentRi_HvacAirTForRowFirstAtVentRi/1
  SdbTxSigProc_Runnable_sys/163(HvacAirTForRowSecAtFlrLe_HvacAirTForRowSecAtFlrLe_write) -> HvacAirTForRowSecAtFlrLe_HvacAirTForRowSecAtFlrLe/1
  SdbTxSigProc_Runnable_sys/164(HvacAirTForRowSecAtFlrRi_HvacAirTForRowSecAtFlrRi_write) -> HvacAirTForRowSecAtFlrRi_HvacAirTForRowSecAtFlrRi/1
  SdbTxSigProc_Runnable_sys/165(HvacAirTForRowSecAtVentLe_HvacAirTForRowSecAtVentLe_write) -> HvacAirTForRowSecAtVentLe_HvacAirTForRowSecAtVentLe/1
  SdbTxSigProc_Runnable_sys/166(HvacAirTForRowSecAtVentRi_HvacAirTForRowSecAtVentRi_write) -> HvacAirTForRowSecAtVentRi_HvacAirTForRowSecAtVentRi/1
  SdbTxSigProc_Runnable_sys/167(HvacCondsorTsp_HvacCondsorTsp_write) -> HvacCondsorTsp_HvacCondsorTsp/1
  SdbTxSigProc_Runnable_sys/168(HvacCoolgEnaFrnt_HvacCoolgEnaFrnt_write) -> HvacCoolgEnaFrnt_HvacCoolgEnaFrnt/1
  SdbTxSigProc_Runnable_sys/169(HvacCoolgEnaRe_HvacCoolgEnaRe_write) -> HvacCoolgEnaRe_HvacCoolgEnaRe/1
  HvacEvaprTSpFrnt_HvacEvaprTSpFrnt_merge/1 -> HvacEvaprTSpFrnt_HvacEvaprTSpFrnt/1
  SdbTxSigProc_Runnable_sys/171(HvacHeatElmAirMFlowEstimd_HvacHeatElmAirMFlowEstimd_write) -> HvacHeatElmAirMFlowEstimd_HvacHeatElmAirMFlowEstimd/1
  SdbTxSigProc_Runnable_sys/172(HvacHeatgReq_HvacHeatgReq_write) -> HvacHeatgReq_HvacHeatgReq/1
  HvacHeatrInletTempReq_HvacHeatrInletTempReq_merge/1 -> HvacHeatrInletTempReq_HvacHeatrInletTempReq/1
  SdbTxSigProc_Runnable_sys/174(HvacRecircAct_HvacRecircAct_write) -> HvacRecircAct_HvacRecircAct/1
  SdbTxSigProc_Runnable_sys/175(HvEgyDesForClima_HvEgyDesForClima_write) -> HvEgyDesForClima_HvEgyDesForClima/1
  SdbTxSigProc_Runnable_sys/176(InPm25SnsrActnReq_InPm25SnsrActnReq_write) -> InPm25SnsrActnReq_InPm25SnsrActnReq/1
  SdbTxSigProc_Runnable_sys/177(IntPm25HiPopUp_IntPm25HiPopUp_write) -> IntPm25HiPopUp_IntPm25HiPopUp/1
  SdbTxSigProc_Runnable_sys/178(LvlOfClimaCmft_LvlOfClimaCmft_write) -> LvlOfClimaCmft_LvlOfClimaCmft/1
  SdbTxSigProc_Runnable_sys/179(PassSeatHeatgPwrAllwd_PassSeatHeatgPwrAllwd_write) -> PassSeatHeatgPwrAllwd_PassSeatHeatgPwrAllwd/1
  SdbTxSigProc_Runnable_sys/180(PassSeatVentnPwrAllwd_PassSeatVentnPwrAllwd_write) -> PassSeatVentnPwrAllwd_PassSeatVentnPwrAllwd/1
  SdbTxSigProc_Runnable_sys/181(PostDrvgClimaAvl_PostDrvgClimaAvl_write) -> PostDrvgClimaAvl_PostDrvgClimaAvl/1
  SdbTxSigProc_Runnable_sys/182(PwrAllwdForElecAirVentn_PwrAllwdForElecAirVentn_write) -> PwrAllwdForElecAirVentn_PwrAllwdForElecAirVentn/1
  SdbTxSigProc_Runnable_sys/183(PwrEstimdAtStbForClima_PwrEstimdAtStbForClima_write) -> PwrEstimdAtStbForClima_PwrEstimdAtStbForClima/1
  SdbTxSigProc_Runnable_sys/184(RlyCmftForClimaReq_RlyCmftForClimaReq_write) -> RlyCmftForClimaReq_RlyCmftForClimaReq/1
  SdbTxSigProc_Runnable_sys/185(RlyCrashForClimaReq_RlyCrashForClimaReq_write) -> RlyCrashForClimaReq_RlyCrashForClimaReq/1
  SdbTxSigProc_Runnable_sys/186(SecRowLeSeatHeatgPwrAllwd_SecRowLeSeatHeatgPwrAllwd_write) -> SecRowLeSeatHeatgPwrAllwd_SecRowLeSeatHeatgPwrAllwd/1
  SdbTxSigProc_Runnable_sys/187(SecRowLeSeatVentnPwrAllwd_SecRowLeSeatVentnPwrAllwd_write) -> SecRowLeSeatVentnPwrAllwd_SecRowLeSeatVentnPwrAllwd/1
  SdbTxSigProc_Runnable_sys/188(SecRowRiSeatHeatgPwrAllwd_SecRowRiSeatHeatgPwrAllwd_write) -> SecRowRiSeatHeatgPwrAllwd_SecRowRiSeatHeatgPwrAllwd/1
  SdbTxSigProc_Runnable_sys/189(SecRowRiSeatVentnPwrAllwd_SecRowRiSeatVentnPwrAllwd_write) -> SecRowRiSeatVentnPwrAllwd_SecRowRiSeatVentnPwrAllwd/1
  SdbTxSigProc_Runnable_sys/190(SteerWhlHeatgDurgClima_SteerWhlHeatgDurgClima_write) -> SteerWhlHeatgDurgClima_SteerWhlHeatgDurgClima/1
  SdbTxSigProc_Runnable_sys/191(TgtHvacAirTForRowFirstLe_TgtHvacAirTForRowFirstLe_write) -> TgtHvacAirTForRowFirstLe_TgtHvacAirTForRowFirstLe/1
  SdbTxSigProc_Runnable_sys/192(TgtHvacAirTForRowFirstRi_TgtHvacAirTForRowFirstRi_write) -> TgtHvacAirTForRowFirstRi_TgtHvacAirTForRowFirstRi/1
  SdbTxSigProc_Runnable_sys/193(TgtHvacAirTForRowSecLe_TgtHvacAirTForRowSecLe_write) -> TgtHvacAirTForRowSecLe_TgtHvacAirTForRowSecLe/1
  SdbTxSigProc_Runnable_sys/194(TgtHvacAirTForRowSecRi_TgtHvacAirTForRowSecRi_write) -> TgtHvacAirTForRowSecRi_TgtHvacAirTForRowSecRi/1
  SdbTxSigProc_Runnable_sys/195(UBoostReqByClima_UBoostReqByClima_write) -> UBoostReqByClima_UBoostReqByClima/1
  SdbTxSigProc_Runnable_sys/196(VehMktForPmSnsr_VehMktForPmSnsr_write) -> VehMktForPmSnsr_VehMktForPmSnsr/1
  SdbTxSigProc_Runnable_sys/197(WindIceDetdFrnt_WindIceDetdFrnt_write) -> WindIceDetdFrnt_WindIceDetdFrnt/1
  SdbTxSigProc_Runnable_sys/198(WindIceDetdRe_WindIceDetdRe_write) -> WindIceDetdRe_WindIceDetdRe/1
  SdbTxSigProc_Runnable_sys/173(HvacHeatrInletTempReq_HvacHeatrInletTempReq_write) -> [HvacHeatrInletTempReq_HvacHeatrInletTempReq_merge_Tag]
  [HvacHeatrInletTempReq_HvacHeatrInletTempReq_merge_Tag] -> HvacHeatrInletTempReq_HvacHeatrInletTempReq_merge/2
  SdbTxSigProc_Init/18(HvacHeatrInletTempReq_HvacHeatrInletTempReq_write) -> [HvacHeatrInletTempReq_HvacHeatrInletTempReq_merge_Tag1]
  [HvacHeatrInletTempReq_HvacHeatrInletTempReq_merge_Tag1] -> HvacHeatrInletTempReq_HvacHeatrInletTempReq_merge/1
  SdbTxSigProc_Runnable_sys/170(HvacEvaprTSpFrnt_HvacEvaprTSpFrnt_write) -> [HvacEvaprTSpFrnt_HvacEvaprTSpFrnt_merge_Tag]
  [HvacEvaprTSpFrnt_HvacEvaprTSpFrnt_merge_Tag] -> HvacEvaprTSpFrnt_HvacEvaprTSpFrnt_merge/2
  SdbTxSigProc_Init/17(HvacEvaprTSpFrnt_HvacEvaprTSpFrnt_write) -> [HvacEvaprTSpFrnt_HvacEvaprTSpFrnt_merge_Tag1]
  [HvacEvaprTSpFrnt_HvacEvaprTSpFrnt_merge_Tag1] -> HvacEvaprTSpFrnt_HvacEvaprTSpFrnt_merge/1
  SdbTxSigProc_Runnable_sys/155(HeatrAirTReq_HeatrAirTReq_write) -> [HeatrAirTReq_HeatrAirTReq_merge_Tag]
  [HeatrAirTReq_HeatrAirTReq_merge_Tag] -> HeatrAirTReq_HeatrAirTReq_merge/2
  SdbTxSigProc_Init/16(HeatrAirTReq_HeatrAirTReq_write) -> [HeatrAirTReq_HeatrAirTReq_merge_Tag1]
  [HeatrAirTReq_HeatrAirTReq_merge_Tag1] -> HeatrAirTReq_HeatrAirTReq_merge/1
  SdbTxSigProc_Runnable_sys/143(CmptmtAirTEstimdExtd_CmptmtAirTEstimdExtd_write) -> [CmptmtAirTEstimdExtd_CmptmtAirTEstimdExtd_merge_Tag]
  [CmptmtAirTEstimdExtd_CmptmtAirTEstimdExtd_merge_Tag] -> CmptmtAirTEstimdExtd_CmptmtAirTEstimdExtd_merge/2
  SdbTxSigProc_Init/15(CmptmtAirTEstimdExtd_CmptmtAirTEstimdExtd_write) -> [CmptmtAirTEstimdExtd_CmptmtAirTEstimdExtd_merge_Tag1]
  [CmptmtAirTEstimdExtd_CmptmtAirTEstimdExtd_merge_Tag1] -> CmptmtAirTEstimdExtd_CmptmtAirTEstimdExtd_merge/1
  SdbTxSigProc_Runnable_sys/142(CmptmtAirTEstimdAtRowFirstLoResl_CmptmtAirTEstimdAtRowFirstLoResl_write) -> [CmptmtAirTEstimdAtRowFirstLoResl_CmptmtAirTEst_fd1601e042567a74]
  [CmptmtAirTEstimdAtRowFirstLoResl_CmptmtAirTEst_fd1601e042567a74] -> CmptmtAirTEstimdAtRowFirstLoResl_CmptmtAirTEstimdAtRowFirstLoResl_merge/2
  SdbTxSigProc_Init/14(CmptmtAirTEstimdAtRowFirstLoResl_CmptmtAirTEstimdAtRowFirstLoResl_write) -> [CmptmtAirTEstimdAtRowFirstLoResl_CmptmtAirTEst_f55b35f4385eaaaa]
  [CmptmtAirTEstimdAtRowFirstLoResl_CmptmtAirTEst_f55b35f4385eaaaa] -> CmptmtAirTEstimdAtRowFirstLoResl_CmptmtAirTEstimdAtRowFirstLoResl_merge/1
  SdbTxSigProc_Runnable_sys/131(CcmCaliSig9_CcmCaliSig9_write) -> [CcmCaliSig9_CcmCaliSig9_merge_Tag]
  [CcmCaliSig9_CcmCaliSig9_merge_Tag] -> CcmCaliSig9_CcmCaliSig9_merge/2
  SdbTxSigProc_Init/13(CcmCaliSig9_CcmCaliSig9_write) -> [CcmCaliSig9_CcmCaliSig9_merge_Tag1]
  [CcmCaliSig9_CcmCaliSig9_merge_Tag1] -> CcmCaliSig9_CcmCaliSig9_merge/1
  SdbTxSigProc_Runnable_sys/130(CcmCaliSig8_CcmCaliSig8_write) -> [CcmCaliSig8_CcmCaliSig8_merge_Tag]
  [CcmCaliSig8_CcmCaliSig8_merge_Tag] -> CcmCaliSig8_CcmCaliSig8_merge/2
  SdbTxSigProc_Init/12(CcmCaliSig8_CcmCaliSig8_write) -> [CcmCaliSig8_CcmCaliSig8_merge_Tag1]
  [CcmCaliSig8_CcmCaliSig8_merge_Tag1] -> CcmCaliSig8_CcmCaliSig8_merge/1
  SdbTxSigProc_Runnable_sys/129(CcmCaliSig7_CcmCaliSig7_write) -> [CcmCaliSig7_CcmCaliSig7_merge_Tag]
  [CcmCaliSig7_CcmCaliSig7_merge_Tag] -> CcmCaliSig7_CcmCaliSig7_merge/2
  SdbTxSigProc_Init/7(CcmCaliSig7_CcmCaliSig7_write) -> [CcmCaliSig7_CcmCaliSig7_merge_Tag1]
  [CcmCaliSig7_CcmCaliSig7_merge_Tag1] -> CcmCaliSig7_CcmCaliSig7_merge/1
  SdbTxSigProc_Runnable_sys/128(CcmCaliSig6_CcmCaliSig6_write) -> [CcmCaliSig6_CcmCaliSig6_merge_Tag]
  [CcmCaliSig6_CcmCaliSig6_merge_Tag] -> CcmCaliSig6_CcmCaliSig6_merge/2
  SdbTxSigProc_Init/6(CcmCaliSig6_CcmCaliSig6_write) -> [CcmCaliSig6_CcmCaliSig6_merge_Tag1]
  [CcmCaliSig6_CcmCaliSig6_merge_Tag1] -> CcmCaliSig6_CcmCaliSig6_merge/1
  SdbTxSigProc_Runnable_sys/127(CcmCaliSig5_CcmCaliSig5_write) -> [CcmCaliSig5_CcmCaliSig5_merge_Tag]
  [CcmCaliSig5_CcmCaliSig5_merge_Tag] -> CcmCaliSig5_CcmCaliSig5_merge/2
  SdbTxSigProc_Init/5(CcmCaliSig5_CcmCaliSig5_write) -> [CcmCaliSig5_CcmCaliSig5_merge_Tag1]
  [CcmCaliSig5_CcmCaliSig5_merge_Tag1] -> CcmCaliSig5_CcmCaliSig5_merge/1
  SdbTxSigProc_Runnable_sys/126(CcmCaliSig4_CcmCaliSig4_write) -> [CcmCaliSig4_CcmCaliSig4_merge_Tag]
  [CcmCaliSig4_CcmCaliSig4_merge_Tag] -> CcmCaliSig4_CcmCaliSig4_merge/2
  SdbTxSigProc_Init/4(CcmCaliSig4_CcmCaliSig4_write) -> [CcmCaliSig4_CcmCaliSig4_merge_Tag1]
  [CcmCaliSig4_CcmCaliSig4_merge_Tag1] -> CcmCaliSig4_CcmCaliSig4_merge/1
  SdbTxSigProc_Runnable_sys/125(CcmCaliSig3_CcmCaliSig3_write) -> [CcmCaliSig3_CcmCaliSig3_merge_Tag]
  [CcmCaliSig3_CcmCaliSig3_merge_Tag] -> CcmCaliSig3_CcmCaliSig3_merge/2
  SdbTxSigProc_Init/3(CcmCaliSig3_CcmCaliSig3_write) -> [CcmCaliSig3_CcmCaliSig3_merge_Tag1]
  [CcmCaliSig3_CcmCaliSig3_merge_Tag1] -> CcmCaliSig3_CcmCaliSig3_merge/1
  SdbTxSigProc_Runnable_sys/124(CcmCaliSig2_CcmCaliSig2_write) -> [CcmCaliSig2_CcmCaliSig2_merge_Tag]
  [CcmCaliSig2_CcmCaliSig2_merge_Tag] -> CcmCaliSig2_CcmCaliSig2_merge/2
  SdbTxSigProc_Init/2(CcmCaliSig2_CcmCaliSig2_write) -> [CcmCaliSig2_CcmCaliSig2_merge_Tag1]
  [CcmCaliSig2_CcmCaliSig2_merge_Tag1] -> CcmCaliSig2_CcmCaliSig2_merge/1
  SdbTxSigProc_Runnable_sys/123(CcmCaliSig12_CcmCaliSig12_write) -> [CcmCaliSig12_CcmCaliSig12_merge_Tag]
  [CcmCaliSig12_CcmCaliSig12_merge_Tag] -> CcmCaliSig12_CcmCaliSig12_merge/2
  SdbTxSigProc_Init/11(CcmCaliSig12_CcmCaliSig12_write) -> [CcmCaliSig12_CcmCaliSig12_merge_Tag1]
  [CcmCaliSig12_CcmCaliSig12_merge_Tag1] -> CcmCaliSig12_CcmCaliSig12_merge/1
  SdbTxSigProc_Runnable_sys/122(CcmCaliSig11_CcmCaliSig11_write) -> [CcmCaliSig11_CcmCaliSig11_merge_Tag]
  [CcmCaliSig11_CcmCaliSig11_merge_Tag] -> CcmCaliSig11_CcmCaliSig11_merge/2
  SdbTxSigProc_Init/10(CcmCaliSig11_CcmCaliSig11_write) -> [CcmCaliSig11_CcmCaliSig11_merge_Tag1]
  [CcmCaliSig11_CcmCaliSig11_merge_Tag1] -> CcmCaliSig11_CcmCaliSig11_merge/1
  SdbTxSigProc_Runnable_sys/121(CcmCaliSig10_CcmCaliSig10_write) -> [CcmCaliSig10_CcmCaliSig10_merge_Tag]
  [CcmCaliSig10_CcmCaliSig10_merge_Tag] -> CcmCaliSig10_CcmCaliSig10_merge/2
  SdbTxSigProc_Init/9(CcmCaliSig10_CcmCaliSig10_write) -> [CcmCaliSig10_CcmCaliSig10_merge_Tag1]
  [CcmCaliSig10_CcmCaliSig10_merge_Tag1] -> CcmCaliSig10_CcmCaliSig10_merge/1
  SdbTxSigProc_Runnable_sys/120(CcmCaliSig1_CcmCaliSig1_write) -> [CcmCaliSig1_CcmCaliSig1_merge_Tag]
  [CcmCaliSig1_CcmCaliSig1_merge_Tag] -> CcmCaliSig1_CcmCaliSig1_merge/2
  SdbTxSigProc_Init/1(CcmCaliSig1_CcmCaliSig1_write) -> [CcmCaliSig1_CcmCaliSig1_merge_Tag1]
  [CcmCaliSig1_CcmCaliSig1_merge_Tag1] -> CcmCaliSig1_CcmCaliSig1_merge/1
  SdbTxSigProc_Runnable_sys/118(AmbTEstimd_AmbTEstimd_write) -> [AmbTEstimd_AmbTEstimd_merge_Tag]
  [AmbTEstimd_AmbTEstimd_merge_Tag] -> AmbTEstimd_AmbTEstimd_merge/2
  SdbTxSigProc_Init/8(AmbTEstimd_AmbTEstimd_write) -> [AmbTEstimd_AmbTEstimd_merge_Tag1]
  [AmbTEstimd_AmbTEstimd_merge_Tag1] -> AmbTEstimd_AmbTEstimd_merge/1
  SdbTxSigProc_Runnable/1 -> SdbTxSigProc_Runnable_sys/Trigger
